simulink model "AcasXuClosedLoop_m_1_batch"  (Simulink 9.0)
settings: Solver=VariableStepAuto  StartTime=0.0  StopTime=20  RelTol=1e-3
values from neighbouring files (symbols this model uses):
  x1_i = randi([-20000,20000])    [Simulate_batch_m_1.m]
  x1_o = randi([-20000,20000])    [Simulate_batch_m_1.m]
  x2_i = randi([-20000,20000])    [Simulate_batch_m_1.m]
  x2_o = randi([-20000,20000])    [Simulate_batch_m_1.m]
  x3_i = rand*2*pi-pi    [Simulate_batch_m_1.m]
  x3_o = rand*2*pi-pi    [Simulate_batch_m_1.m]
libraries: neural x1560
(part 4 of 60)

== system AcasXuClosedLoop_m_1_batch/networks/AcasXu_4_1/Layer 1 ==
  "p{1}" : Inport  PortDimensions=5
      mask Display: e = 0.06;c1 = [120 230 180]/255;c2 = ([120 230 180]+0)/(256+128);c3 = ([120 230 180]+128)/(256+128);patch([0 1 1 0],[0 0 1 1],c1);patch([0 1 1 1-e 1-e 0+e],[0 0 1 1-e 0+e 0+e],c2);patch([0 0 1 1-e 0+e 0+e],[0 1 1 1-e 1-e 0+e],c3);plot([0 1 1 0 0],[0 0 1 1 0]);
  Delays 1 : Subsystem
      mask Display: e = 0.06;c1 = [120 150 230]/255;c2 = ([120 150 230]+0)/383;c3 = ([120 150 230]+128)/383;patch([0 1 1 0],[0 0 1 1],c1);patch([0 1 1 1-e 1-e 0+e],[0 0 1 1-e 0+e 0+e],c2);patch([0 0 1 1-e 0+e 0+e],[0 1 1 1-e 1-e 0+e],c3);plot([0 1 1 0 0],[0 0 1 1 0]);disp('0');
  "IW{1,1}" : Subsystem
      mask Display: e = 0.06;c1 = [120 150 230]/255;c2 = ([120 150 230]+0)/383;c3 = ([120 150 230]+128)/383;patch([0 1 1 0],[0 0 1 1],c1);patch([0 1 1 1-e 1-e 0+e],[0 0 1 1-e 0+e 0+e],c2);patch([0 0 1 1-e 0+e 0+e],[0 1 1 1-e 1-e 0+e],c3);plot([0 1 1 0 0],[0 0 1 1 0]);disp('W');
  "b{1}" : Constant  Value=[0.1348229999999999983995024877003743313252925872802734375;-0.0238180999999999983007814563507054117508232593536376953125;-0.11955000000000000348610029732299153693020343780517578125;-0.380141000000000006675549002466141246259212493896484375;-0.453220000000000011741718708435655571520328521728515625;-0.7901599999999999734967559561482630670070648193359375;-0.59201899999999996193622564533143304288387298583984375;-0.060997400000000000230837571280062547884881496429443359375;0.041191899999999996573318838954946841113269329071044921875;0.138994000000000006433964472307707183063030242919921875;0.5230390000000000316759951601852662861347198486328125;0.1683389999999999886437507257141987793147563934326171875;-0.2097199999999999897593028208575560711324214935302734375;-0.30047699999999999409538986583356745541095733642578125;-0.6601470000000000393214349969639442861080169677734375;0.33775800000000000267164068645797669887542724609375;-0.72938300000000000355981910615810193121433258056640625;-0.354287999999999991818100397722446359694004058837890625;0.330224000000000017518431150165270082652568817138671875;-0.01135309999999999965025754278258318663574755191802978515625;-0.0360036999999999995925037410415825434029102325439453125;-0.0244249999999999987954080182817051536403596401214599609375;0.2328119999999999911732828650201554410159587860107421875;0.1819800000000000028688162956314045004546642303466796875;0.03522630000000000205506722750214976258575916290283203125;0.45888200000000001210764821735210716724395751953125;-0.178321000000000007279510327862226404249668121337890625;0.055081100000000000893596308060295996256172657012939453125;-0.01507080000000000051529891464952015667222440242767333984375;-0.4580159999999999786979287819121964275836944580078125;-0.0500089999999999978985698589895037002861499786376953125;0.06709189999999999598490063590361387468874454498291015625;-0.323990999999999973457676105681457556784152984619140625;0.233887000000000011556977597138029523193836212158203125;0.1795219999999999871409528395815868861973285675048828125;-0.47103499999999998149036173344939015805721282958984375;0.11780400000000000593214366517713642679154872894287109375;-0.1605830000000000035154101851730956695973873138427734375;-0.02595340000000000146851419913218705914914608001708984375;-0.19649199999999999999289457264239899814128875732421875;0.09367630000000000400905975084242527373135089874267578125;-0.264062999999999992173371765602496452629566192626953125;-0.350741000000000024972024448288721032440662384033203125;-0.68448200000000003484501576167531311511993408203125;-0.2153859999999999941255879321033717133104801177978515625;0.60005299999999994753352439147420227527618408203125;0.276144000000000000571986902286880649626255035400390625;-0.57574000000000002952305067083216272294521331787109375;-0.5823739999999999472635181518853642046451568603515625;0.1123949999999999949107376551182824186980724334716796875]
      mask Display: e = 0.06;c1 = [120 150 230]/255;c2 = ([120 150 230]+0)/383;c3 = ([120 150 230]+128)/383;patch([0 1 1 0],[0 0 1 1],c1);patch([0 1 1 1-e 1-e 0+e],[0 0 1 1-e 0+e 0+e],c2);patch([0 0 1 1-e 0+e 0+e],[0 1 1 1-e 1-e 0+e],c3);plot([0 1 1 0 0],[0 0 1 1 0]);disp('b');
  netsum : Sum  Inputs=2  SaturateOnIntegerOverflow=on  InputSameDT=on  OutDataTypeStr=Inherit: Same as first input
      mask Display: e = 0.06;c1 = [120 150 230]/255;c2 = ([120 150 230]+0)/383;c3 = ([120 150 230]+128)/383;patch([0 1 1 0],[0 0 1 1],c1);patch([0 1 1 1-e 1-e 0+e],[0 0 1 1-e 0+e 0+e],c2);patch([0 0 1 1-e 0+e 0+e],[0 1 1 1-e 1-e 0+e],c3);plot([0 1 1 0 0],[0 0 1 1 0]);disp('+');
  poslin : neural/Transfer Functions/poslin
      settings: SourceProductName=Deep Learning Toolbox  SourceProductBaseCode=NN
  "a{1}" : Outport  InitialOutput=[0;0;0;0;0;0;0;0;0;0;0;0;0;0;0;0;0;0;0;0;0;0;0;0;0;0;0;0;0;0;0;0;0;0;0;0;0;0;0;0;0;0;0;0;0;0;0;0;0;0]
      mask Display: e = 0.06;c1 = [120 230 180]/255;c2 = ([120 230 180]+0)/(256+128);c3 = ([120 230 180]+128)/(256+128);patch([0 1 1 0],[0 0 1 1],c1);patch([0 1 1 1-e 1-e 0+e],[0 0 1 1-e 0+e 0+e],c2);patch([0 0 1 1-e 0+e 0+e],[0 1 1 1-e 1-e 0+e],c3);plot([0 1 1 0 0],[0 0 1 1 0]);
  wires:
  poslin/1 -> "a{1}"/1
  netsum/1 -> poslin/1
  "b{1}"/1 -> netsum/2
  "IW{1,1}"/1("iz{1,1}") -> netsum/1
  Delays 1/1("pd{1,1}") -> "IW{1,1}"/1("pd{1,1}")
  "p{1}"/1 -> Delays 1/1("p{1}")

== system AcasXuClosedLoop_m_1_batch/networks/AcasXu_4_1/Layer 2 ==
  "a{1} " : Inport  PortDimensions=50
      mask Display: e = 0.06;c1 = [120 230 180]/255;c2 = ([120 230 180]+0)/(256+128);c3 = ([120 230 180]+128)/(256+128);patch([0 1 1 0],[0 0 1 1],c1);patch([0 1 1 1-e 1-e 0+e],[0 0 1 1-e 0+e 0+e],c2);patch([0 0 1 1-e 0+e 0+e],[0 1 1 1-e 1-e 0+e],c3);plot([0 1 1 0 0],[0 0 1 1 0]);
  Delays 1 : Subsystem
      mask Display: e = 0.06;c1 = [120 150 230]/255;c2 = ([120 150 230]+0)/383;c3 = ([120 150 230]+128)/383;patch([0 1 1 0],[0 0 1 1],c1);patch([0 1 1 1-e 1-e 0+e],[0 0 1 1-e 0+e 0+e],c2);patch([0 0 1 1-e 0+e 0+e],[0 1 1 1-e 1-e 0+e],c3);plot([0 1 1 0 0],[0 0 1 1 0]);disp('0');
  "LW{2,1}" : Subsystem
      mask Display: e = 0.06;c1 = [120 150 230]/255;c2 = ([120 150 230]+0)/383;c3 = ([120 150 230]+128)/383;patch([0 1 1 0],[0 0 1 1],c1);patch([0 1 1 1-e 1-e 0+e],[0 0 1 1-e 0+e 0+e],c2);patch([0 0 1 1-e 0+e 0+e],[0 1 1 1-e 1-e 0+e],c3);plot([0 1 1 0 0],[0 0 1 1 0]);disp('W');
  "b{2}" : Constant  Value=[0.432821999999999984520826501466217450797557830810546875;-1.259370000000000100470742836478166282176971435546875;-0.45713700000000001555378048578859306871891021728515625;-0.65248300000000003517897084748256020247936248779296875;0.0116892000000000002846167745929051307030022144317626953125;0.9686099999999999710098563809879124164581298828125;-0.00109700000000000010176581799470341138658113777637481689453125;-0.01449650000000000070465855372958685620687901973724365234375;-0.01553530000000000015070167336261874879710376262664794921875;-0.1711300000000000043343106881366111338138580322265625;1.346410000000000106723518911167047917842864990234375;-0.0236382000000000015715873047383865923620760440826416015625;1.136740000000000083701934272539801895618438720703125;-0.98688699999999995871036162498057819902896881103515625;-0.202730999999999994543031789362430572509765625;0.28883599999999998164668113531661219894886016845703125;-0.18953900000000001302424834648263640701770782470703125;-0.67643200000000003324629460621508769690990447998046875;-0.77303200000000005243094847173779271543025970458984375;1.2642599999999999393907046396634541451930999755859375;-0.51136499999999995846877709482214413583278656005859375;1.3603799999999999226218960757250897586345672607421875;-0.0432717999999999991533883303418406285345554351806640625;-0.273573999999999983856469043530523777008056640625;0.3089749999999999996447286321199499070644378662109375;-0.05264359999999999872866141004124074243009090423583984375;1.1708000000000000628830321147688664495944976806640625;-0.01064329999999999963600227914639617665670812129974365234375;-0.50644199999999994776800349427503533661365509033203125;0.1564000000000000112354570092065841890871524810791015625;0.1855920000000000069650951672883820720016956329345703125;-0.0237028999999999989700683045157347805798053741455078125;-0.95172800000000001841016228354419581592082977294921875;-0.322857000000000005091038701721117831766605377197265625;-0.382485000000000019415580254644737578928470611572265625;-0.1345760000000000011777245845223660580813884735107421875;-0.01455150000000000019839685450051547377370297908782958984375;-0.269760999999999973031350464225397445261478424072265625;-0.88523399999999996534683077697991393506526947021484375;9.9680700000000002614607141904201625948189757764339447021484375e-05;-0.0100038999999999995982324918486483511514961719512939453125;1.2512399999999999078426071719150058925151824951171875;0.171111999999999986332710477654472924768924713134765625;-0.409106000000000025185187269016751088201999664306640625;-0.6994439999999999546531626037904061377048492431640625;0.444589999999999985202947527795913629233837127685546875;0.535841000000000011738165994756855070590972900390625;0.04694199999999999761701729994456400163471698760986328125;0.2113869999999999915729631538852117955684661865234375;0.58456699999999994776800349427503533661365509033203125]
      mask Display: e = 0.06;c1 = [120 150 230]/255;c2 = ([120 150 230]+0)/383;c3 = ([120 150 230]+128)/383;patch([0 1 1 0],[0 0 1 1],c1);patch([0 1 1 1-e 1-e 0+e],[0 0 1 1-e 0+e 0+e],c2);patch([0 0 1 1-e 0+e 0+e],[0 1 1 1-e 1-e 0+e],c3);plot([0 1 1 0 0],[0 0 1 1 0]);disp('b');
  netsum : Sum  Inputs=2  SaturateOnIntegerOverflow=on  InputSameDT=on  OutDataTypeStr=Inherit: Same as first input
      mask Display: e = 0.06;c1 = [120 150 230]/255;c2 = ([120 150 230]+0)/383;c3 = ([120 150 230]+128)/383;patch([0 1 1 0],[0 0 1 1],c1);patch([0 1 1 1-e 1-e 0+e],[0 0 1 1-e 0+e 0+e],c2);patch([0 0 1 1-e 0+e 0+e],[0 1 1 1-e 1-e 0+e],c3);plot([0 1 1 0 0],[0 0 1 1 0]);disp('+');
  poslin : neural/Transfer Functions/poslin
      settings: SourceProductName=Deep Learning Toolbox  SourceProductBaseCode=NN
  "a{2}" : Outport  InitialOutput=[0;0;0;0;0;0;0;0;0;0;0;0;0;0;0;0;0;0;0;0;0;0;0;0;0;0;0;0;0;0;0;0;0;0;0;0;0;0;0;0;0;0;0;0;0;0;0;0;0;0]
      mask Display: e = 0.06;c1 = [120 230 180]/255;c2 = ([120 230 180]+0)/(256+128);c3 = ([120 230 180]+128)/(256+128);patch([0 1 1 0],[0 0 1 1],c1);patch([0 1 1 1-e 1-e 0+e],[0 0 1 1-e 0+e 0+e],c2);patch([0 0 1 1-e 0+e 0+e],[0 1 1 1-e 1-e 0+e],c3);plot([0 1 1 0 0],[0 0 1 1 0]);
  wires:
  poslin/1 -> "a{2}"/1
  netsum/1 -> poslin/1
  "b{2}"/1 -> netsum/2
  "LW{2,1}"/1("lz{2,1}") -> netsum/1
  Delays 1/1("pd{2,1}") -> "LW{2,1}"/1("ad{2,1}")
  "a{1} "/1 -> Delays 1/1("p{2}")

== system AcasXuClosedLoop_m_1_batch/networks/AcasXu_4_1/Layer 3 ==
  "a{2} " : Inport  PortDimensions=50
      mask Display: e = 0.06;c1 = [120 230 180]/255;c2 = ([120 230 180]+0)/(256+128);c3 = ([120 230 180]+128)/(256+128);patch([0 1 1 0],[0 0 1 1],c1);patch([0 1 1 1-e 1-e 0+e],[0 0 1 1-e 0+e 0+e],c2);patch([0 0 1 1-e 0+e 0+e],[0 1 1 1-e 1-e 0+e],c3);plot([0 1 1 0 0],[0 0 1 1 0]);
  Delays 1 : Subsystem
      mask Display: e = 0.06;c1 = [120 150 230]/255;c2 = ([120 150 230]+0)/383;c3 = ([120 150 230]+128)/383;patch([0 1 1 0],[0 0 1 1],c1);patch([0 1 1 1-e 1-e 0+e],[0 0 1 1-e 0+e 0+e],c2);patch([0 0 1 1-e 0+e 0+e],[0 1 1 1-e 1-e 0+e],c3);plot([0 1 1 0 0],[0 0 1 1 0]);disp('0');
  "LW{3,2}" : Subsystem
      mask Display: e = 0.06;c1 = [120 150 230]/255;c2 = ([120 150 230]+0)/383;c3 = ([120 150 230]+128)/383;patch([0 1 1 0],[0 0 1 1],c1);patch([0 1 1 1-e 1-e 0+e],[0 0 1 1-e 0+e 0+e],c2);patch([0 0 1 1-e 0+e 0+e],[0 1 1 1-e 1-e 0+e],c3);plot([0 1 1 0 0],[0 0 1 1 0]);disp('W');
  "b{3}" : Constant  Value=[0.75966199999999994840749195645912550389766693115234375;-0.838961999999999985533349899924360215663909912109375;-1.1200300000000000810729261502274312078952789306640625;-0.51097300000000001052313791660708375275135040283203125;-0.135124999999999995115018691649311222136020660400390625;-0.82995099999999999429434183184639550745487213134765625;-0.7141610000000000457731630376656539738178253173828125;1.510499999999999953814722175593487918376922607421875;0.282934999999999992059684927880880422890186309814453125;-0.294370999999999993779198348420322872698307037353515625;-0.455872999999999972686737237381748855113983154296875;-0.7350980000000000291748847303097136318683624267578125;-0.36855599999999999472066747330245561897754669189453125;-0.141824000000000005616840326183591969311237335205078125;-0.9845960000000000267306177192949689924716949462890625;-0.1444709999999999883169010672645526938140392303466796875;0.63247799999999998465938233493943698704242706298828125;-0.6318770000000000219841922444174997508525848388671875;-0.00854197999999999936504568864847897202707827091217041015625;0.61706099999999997063326873103505931794643402099609375;-1.305309999999999970299313645227812230587005615234375;0.65445100000000000495248286824789829552173614501953125;-0.8968920000000000225526264330255798995494842529296875;0.022811299999999999632560587770058191381394863128662109375;-0.347494000000000025085711286010337062180042266845703125;-0.138658000000000003471001264188089407980442047119140625;-0.81998499999999996390442902338691055774688720703125;-1.0180700000000000304822833641082979738712310791015625;-0.88269600000000003614530896811629645526409149169921875;-1.585620000000000029416469260468147695064544677734375;-1.1131299999999999528199623455293476581573486328125;-0.888298999999999949750417727045714855194091796875;-1.0311099999999999710098563809879124164581298828125;-0.48253800000000002246025587737676687538623809814453125;-0.363474000000000019294787989565520547330379486083984375;-0.670150000000000023447910280083306133747100830078125;-0.645361999999999991217691786005161702632904052734375;0.36204300000000000370192765331012196838855743408203125;-1.3692699999999999871391764827421866357326507568359375;-2.15331999999999990080823408789001405239105224609375;0.321091999999999988535392958510783500969409942626953125;1.4917700000000000404298816647497005760669708251953125;0.1303020000000000011564083024495630525052547454833984375;-0.7060370000000000256790144703700207173824310302734375;1.1547400000000000996891458271420560777187347412109375;-0.73546699999999998187405481075984425842761993408203125;-0.207056999999999991057819670459139160811901092529296875;-0.95738800000000001677591399129596538841724395751953125;-1.3000700000000000589039927945123054087162017822265625;-0.1655649999999999899547020731915836222469806671142578125]
      mask Display: e = 0.06;c1 = [120 150 230]/255;c2 = ([120 150 230]+0)/383;c3 = ([120 150 230]+128)/383;patch([0 1 1 0],[0 0 1 1],c1);patch([0 1 1 1-e 1-e 0+e],[0 0 1 1-e 0+e 0+e],c2);patch([0 0 1 1-e 0+e 0+e],[0 1 1 1-e 1-e 0+e],c3);plot([0 1 1 0 0],[0 0 1 1 0]);disp('b');
  netsum : Sum  Inputs=2  SaturateOnIntegerOverflow=on  InputSameDT=on  OutDataTypeStr=Inherit: Same as first input
      mask Display: e = 0.06;c1 = [120 150 230]/255;c2 = ([120 150 230]+0)/383;c3 = ([120 150 230]+128)/383;patch([0 1 1 0],[0 0 1 1],c1);patch([0 1 1 1-e 1-e 0+e],[0 0 1 1-e 0+e 0+e],c2);patch([0 0 1 1-e 0+e 0+e],[0 1 1 1-e 1-e 0+e],c3);plot([0 1 1 0 0],[0 0 1 1 0]);disp('+');
  poslin : neural/Transfer Functions/poslin
      settings: SourceProductName=Deep Learning Toolbox  SourceProductBaseCode=NN
  "a{3}" : Outport  InitialOutput=[0;0;0;0;0;0;0;0;0;0;0;0;0;0;0;0;0;0;0;0;0;0;0;0;0;0;0;0;0;0;0;0;0;0;0;0;0;0;0;0;0;0;0;0;0;0;0;0;0;0]
      mask Display: e = 0.06;c1 = [120 230 180]/255;c2 = ([120 230 180]+0)/(256+128);c3 = ([120 230 180]+128)/(256+128);patch([0 1 1 0],[0 0 1 1],c1);patch([0 1 1 1-e 1-e 0+e],[0 0 1 1-e 0+e 0+e],c2);patch([0 0 1 1-e 0+e 0+e],[0 1 1 1-e 1-e 0+e],c3);plot([0 1 1 0 0],[0 0 1 1 0]);
  wires:
  poslin/1 -> "a{3}"/1
  netsum/1 -> poslin/1
  "b{3}"/1 -> netsum/2
  "LW{3,2}"/1("lz{3,2}") -> netsum/1
  Delays 1/1("pd{3,2}") -> "LW{3,2}"/1("ad{3,2}")
  "a{2} "/1 -> Delays 1/1("p{3}")

== system AcasXuClosedLoop_m_1_batch/networks/AcasXu_4_1/Layer 4 ==
  "a{3} " : Inport  PortDimensions=50
      mask Display: e = 0.06;c1 = [120 230 180]/255;c2 = ([120 230 180]+0)/(256+128);c3 = ([120 230 180]+128)/(256+128);patch([0 1 1 0],[0 0 1 1],c1);patch([0 1 1 1-e 1-e 0+e],[0 0 1 1-e 0+e 0+e],c2);patch([0 0 1 1-e 0+e 0+e],[0 1 1 1-e 1-e 0+e],c3);plot([0 1 1 0 0],[0 0 1 1 0]);
  Delays 1 : Subsystem
      mask Display: e = 0.06;c1 = [120 150 230]/255;c2 = ([120 150 230]+0)/383;c3 = ([120 150 230]+128)/383;patch([0 1 1 0],[0 0 1 1],c1);patch([0 1 1 1-e 1-e 0+e],[0 0 1 1-e 0+e 0+e],c2);patch([0 0 1 1-e 0+e 0+e],[0 1 1 1-e 1-e 0+e],c3);plot([0 1 1 0 0],[0 0 1 1 0]);disp('0');
  "LW{4,3}" : Subsystem
      mask Display: e = 0.06;c1 = [120 150 230]/255;c2 = ([120 150 230]+0)/383;c3 = ([120 150 230]+128)/383;patch([0 1 1 0],[0 0 1 1],c1);patch([0 1 1 1-e 1-e 0+e],[0 0 1 1-e 0+e 0+e],c2);patch([0 0 1 1-e 0+e 0+e],[0 1 1 1-e 1-e 0+e],c3);plot([0 1 1 0 0],[0 0 1 1 0]);disp('W');
  "b{4}" : Constant  Value=[-0.6820079999999999476045786650502122938632965087890625;-3.08436999999999983401721692644059658050537109375;0.585555999999999965410779623198322951793670654296875;-0.5398359999999999825348595550167374312877655029296875;-0.55025299999999999212008106042048893868923187255859375;0.0256048000000000004761080418802521307952702045440673828125;-1.7290099999999999358379909608629532158374786376953125;-0.1298610000000000042064129956997931003570556640625;-0.8305789999999999562163566224626265466213226318359375;-0.196811999999999986954435371444560587406158447265625;-0.6160639999999999449897813974530436098575592041015625;0.3433990000000000097912788987741805613040924072265625;-1.597210000000000018616219676914624869823455810546875;0.1576450000000000073452355309200356714427471160888671875;0.88317800000000001858779796748422086238861083984375;-0.70011999999999996457944462235900573432445526123046875;-0.286934000000000022367885321727953851222991943359375;0.637162000000000006139089236967265605926513671875;1.2516700000000000603250782660325057804584503173828125;-0.01162849999999999994759747323769261129200458526611328125;-0.5578469999999999817674734003958292305469512939453125;-1.00337999999999993860910763032734394073486328125;1.05571999999999999175770426518283784389495849609375;-1.24138999999999999346300683100707828998565673828125;0.186377999999999988123278171769925393164157867431640625;0.0017374199999999999366462333227900671772658824920654296875;0.105161000000000004472866521609830670058727264404296875;0.54805800000000004512656914812396280467510223388671875;0.08856619999999999770512459917881642468273639678955078125;0.1579730000000000023074875343809253536164760589599609375;-0.0757977999999999985103471544789499603211879730224609375;1.2585900000000000975575176198617555201053619384765625;-1.461959999999999926245664028101600706577301025390625;0.62902299999999999879918277656543068587779998779296875;-0.7479839999999999822222207512822933495044708251953125;0.09964230000000000309068326487249578349292278289794921875;1.006110000000000059827698351000435650348663330078125;0.77711699999999994670218939063488505780696868896484375;0.325390000000000012558842854559770785272121429443359375;-1.7636499999999999399591388282715342938899993896484375;-0.53327899999999994751220810940139926970005035400390625;-0.77692799999999995197441648997482843697071075439453125;-0.73104299999999999837285713510937057435512542724609375;0.64756599999999997496757941917167045176029205322265625;-0.1434709999999999874287226475644274614751338958740234375;0.751273999999999997356781022972427308559417724609375;0.4473059999999999813979911778005771338939666748046875;1.2945800000000000640199004919850267469882965087890625;-0.55212600000000000566302560400799848139286041259765625;1.038089999999999957225327307241968810558319091796875]
      mask Display: e = 0.06;c1 = [120 150 230]/255;c2 = ([120 150 230]+0)/383;c3 = ([120 150 230]+128)/383;patch([0 1 1 0],[0 0 1 1],c1);patch([0 1 1 1-e 1-e 0+e],[0 0 1 1-e 0+e 0+e],c2);patch([0 0 1 1-e 0+e 0+e],[0 1 1 1-e 1-e 0+e],c3);plot([0 1 1 0 0],[0 0 1 1 0]);disp('b');
  netsum : Sum  Inputs=2  SaturateOnIntegerOverflow=on  InputSameDT=on  OutDataTypeStr=Inherit: Same as first input
      mask Display: e = 0.06;c1 = [120 150 230]/255;c2 = ([120 150 230]+0)/383;c3 = ([120 150 230]+128)/383;patch([0 1 1 0],[0 0 1 1],c1);patch([0 1 1 1-e 1-e 0+e],[0 0 1 1-e 0+e 0+e],c2);patch([0 0 1 1-e 0+e 0+e],[0 1 1 1-e 1-e 0+e],c3);plot([0 1 1 0 0],[0 0 1 1 0]);disp('+');
  poslin : neural/Transfer Functions/poslin
      settings: SourceProductName=Deep Learning Toolbox  SourceProductBaseCode=NN
  "a{4}" : Outport  InitialOutput=[0;0;0;0;0;0;0;0;0;0;0;0;0;0;0;0;0;0;0;0;0;0;0;0;0;0;0;0;0;0;0;0;0;0;0;0;0;0;0;0;0;0;0;0;0;0;0;0;0;0]
      mask Display: e = 0.06;c1 = [120 230 180]/255;c2 = ([120 230 180]+0)/(256+128);c3 = ([120 230 180]+128)/(256+128);patch([0 1 1 0],[0 0 1 1],c1);patch([0 1 1 1-e 1-e 0+e],[0 0 1 1-e 0+e 0+e],c2);patch([0 0 1 1-e 0+e 0+e],[0 1 1 1-e 1-e 0+e],c3);plot([0 1 1 0 0],[0 0 1 1 0]);
  wires:
  poslin/1 -> "a{4}"/1
  netsum/1 -> poslin/1
  "b{4}"/1 -> netsum/2
  "LW{4,3}"/1("lz{4,3}") -> netsum/1
  Delays 1/1("pd{4,3}") -> "LW{4,3}"/1("ad{4,3}")
  "a{3} "/1 -> Delays 1/1("p{4}")

== system AcasXuClosedLoop_m_1_batch/networks/AcasXu_4_1/Layer 5 ==
  "a{4} " : Inport  PortDimensions=50
      mask Display: e = 0.06;c1 = [120 230 180]/255;c2 = ([120 230 180]+0)/(256+128);c3 = ([120 230 180]+128)/(256+128);patch([0 1 1 0],[0 0 1 1],c1);patch([0 1 1 1-e 1-e 0+e],[0 0 1 1-e 0+e 0+e],c2);patch([0 0 1 1-e 0+e 0+e],[0 1 1 1-e 1-e 0+e],c3);plot([0 1 1 0 0],[0 0 1 1 0]);
  Delays 1 : Subsystem
      mask Display: e = 0.06;c1 = [120 150 230]/255;c2 = ([120 150 230]+0)/383;c3 = ([120 150 230]+128)/383;patch([0 1 1 0],[0 0 1 1],c1);patch([0 1 1 1-e 1-e 0+e],[0 0 1 1-e 0+e 0+e],c2);patch([0 0 1 1-e 0+e 0+e],[0 1 1 1-e 1-e 0+e],c3);plot([0 1 1 0 0],[0 0 1 1 0]);disp('0');
  "LW{5,4}" : Subsystem
      mask Display: e = 0.06;c1 = [120 150 230]/255;c2 = ([120 150 230]+0)/383;c3 = ([120 150 230]+128)/383;patch([0 1 1 0],[0 0 1 1],c1);patch([0 1 1 1-e 1-e 0+e],[0 0 1 1-e 0+e 0+e],c2);patch([0 0 1 1-e 0+e 0+e],[0 1 1 1-e 1-e 0+e],c3);plot([0 1 1 0 0],[0 0 1 1 0]);disp('W');
  "b{5}" : Constant  Value=[-1.148800000000000043343106881366111338138580322265625;-1.268780000000000018900436771218664944171905517578125;-0.448139999999999982804865794605575501918792724609375;0.4046790000000000109281472759903408586978912353515625;1.01714999999999999857891452847979962825775146484375;0.305279000000000022563284574061981402337551116943359375;0.08392149999999999610000855909675010479986667633056640625;0.52215100000000003177547114319168031215667724609375;-1.074980000000000046611603465862572193145751953125;-1.4273800000000000931521526581491343677043914794921875;0.52887099999999998001243284306838177144527435302734375;-0.284766999999999992354560163221322000026702880859375;0.462851999999999985657694878682377748191356658935546875;-1.434930000000000038795633372501470148563385009765625;1.021269999999999900097691352129913866519927978515625;1.4297100000000000363797880709171295166015625;-0.55277399999999998758681840627104975283145904541015625;-1.43315999999999998948396751075051724910736083984375;0.64438099999999998157562686174060218036174774169921875;1.3235900000000000442668124378542415797710418701171875;-0.356341000000000018843593352357856929302215576171875;-1.3253399999999999625543978254427202045917510986328125;0.47899700000000000610356210017926059663295745849609375;0.09044280000000000374615893861118820495903491973876953125;-0.1897939999999999904911618386904592625796794891357421875;0.081688700000000002976463520099059678614139556884765625;-0.1393810000000000048903103788688895292580127716064453125;0.79328699999999996439470351106137968599796295166015625;0.853005000000000013216094885137863457202911376953125;0.8116689999999999738378164693131111562252044677734375;0.1342210000000000069686478809671825729310512542724609375;-0.89552500000000001545430450278217904269695281982421875;0.41882399999999997408650642682914622128009796142578125;0.5555499999999999882760448599583469331264495849609375;0.2302760000000000084607876260633929632604122161865234375;1.2937499999999999555910790149937383830547332763671875;0.65880199999999999871391764827421866357326507568359375;0.387861999999999984556353638254222460091114044189453125;-0.1655999999999999972022379779446055181324481964111328125;1.0075700000000000766675611885148100554943084716796875;0.194539999999999990709653729936690069735050201416015625;-1.03254000000000001335820343228988349437713623046875;-0.144120999999999999108268866621074266731739044189453125;0.42739700000000002688693712116219103336334228515625;-1.1556599999999999095479097377392463386058807373046875;1.261789999999999967172925607883371412754058837890625;-0.282252000000000002888356220864807255566120147705078125;-0.00985550999999999961798113901068063569255173206329345703125;0.88892300000000001869437937784823589026927947998046875;2.444459999999999855191390452091582119464874267578125]
      mask Display: e = 0.06;c1 = [120 150 230]/255;c2 = ([120 150 230]+0)/383;c3 = ([120 150 230]+128)/383;patch([0 1 1 0],[0 0 1 1],c1);patch([0 1 1 1-e 1-e 0+e],[0 0 1 1-e 0+e 0+e],c2);patch([0 0 1 1-e 0+e 0+e],[0 1 1 1-e 1-e 0+e],c3);plot([0 1 1 0 0],[0 0 1 1 0]);disp('b');
  netsum : Sum  Inputs=2  SaturateOnIntegerOverflow=on  InputSameDT=on  OutDataTypeStr=Inherit: Same as first input
      mask Display: e = 0.06;c1 = [120 150 230]/255;c2 = ([120 150 230]+0)/383;c3 = ([120 150 230]+128)/383;patch([0 1 1 0],[0 0 1 1],c1);patch([0 1 1 1-e 1-e 0+e],[0 0 1 1-e 0+e 0+e],c2);patch([0 0 1 1-e 0+e 0+e],[0 1 1 1-e 1-e 0+e],c3);plot([0 1 1 0 0],[0 0 1 1 0]);disp('+');
  poslin : neural/Transfer Functions/poslin
      settings: SourceProductName=Deep Learning Toolbox  SourceProductBaseCode=NN
  "a{5}" : Outport  InitialOutput=[0;0;0;0;0;0;0;0;0;0;0;0;0;0;0;0;0;0;0;0;0;0;0;0;0;0;0;0;0;0;0;0;0;0;0;0;0;0;0;0;0;0;0;0;0;0;0;0;0;0]
      mask Display: e = 0.06;c1 = [120 230 180]/255;c2 = ([120 230 180]+0)/(256+128);c3 = ([120 230 180]+128)/(256+128);patch([0 1 1 0],[0 0 1 1],c1);patch([0 1 1 1-e 1-e 0+e],[0 0 1 1-e 0+e 0+e],c2);patch([0 0 1 1-e 0+e 0+e],[0 1 1 1-e 1-e 0+e],c3);plot([0 1 1 0 0],[0 0 1 1 0]);
  wires:
  poslin/1 -> "a{5}"/1
  netsum/1 -> poslin/1
  "b{5}"/1 -> netsum/2
  "LW{5,4}"/1("lz{5,4}") -> netsum/1
  Delays 1/1("pd{5,4}") -> "LW{5,4}"/1("ad{5,4}")
  "a{4} "/1 -> Delays 1/1("p{5}")

== system AcasXuClosedLoop_m_1_batch/networks/AcasXu_4_1/Layer 6 ==
  "a{5} " : Inport  PortDimensions=50
      mask Display: e = 0.06;c1 = [120 230 180]/255;c2 = ([120 230 180]+0)/(256+128);c3 = ([120 230 180]+128)/(256+128);patch([0 1 1 0],[0 0 1 1],c1);patch([0 1 1 1-e 1-e 0+e],[0 0 1 1-e 0+e 0+e],c2);patch([0 0 1 1-e 0+e 0+e],[0 1 1 1-e 1-e 0+e],c3);plot([0 1 1 0 0],[0 0 1 1 0]);
  Delays 1 : Subsystem
      mask Display: e = 0.06;c1 = [120 150 230]/255;c2 = ([120 150 230]+0)/383;c3 = ([120 150 230]+128)/383;patch([0 1 1 0],[0 0 1 1],c1);patch([0 1 1 1-e 1-e 0+e],[0 0 1 1-e 0+e 0+e],c2);patch([0 0 1 1-e 0+e 0+e],[0 1 1 1-e 1-e 0+e],c3);plot([0 1 1 0 0],[0 0 1 1 0]);disp('0');
  "LW{6,5}" : Subsystem
      mask Display: e = 0.06;c1 = [120 150 230]/255;c2 = ([120 150 230]+0)/383;c3 = ([120 150 230]+128)/383;patch([0 1 1 0],[0 0 1 1],c1);patch([0 1 1 1-e 1-e 0+e],[0 0 1 1-e 0+e 0+e],c2);patch([0 0 1 1-e 0+e 0+e],[0 1 1 1-e 1-e 0+e],c3);plot([0 1 1 0 0],[0 0 1 1 0]);disp('W');
  "b{6}" : Constant  Value=[-2.6473800000000000665068000671453773975372314453125;-0.10213899999999999368416325751240947283804416656494140625;0.09831600000000000061373128801278653554618358612060546875;0.2058689999999999964419572506812983192503452301025390625;-0.0282583999999999994912069922747832606546580791473388671875;0.196272000000000002017941369558684527873992919921875;-0.4957759999999999944719775157864205539226531982421875;-0.031972300000000002107025665054607088677585124969482421875;0.03432000000000000328181926079196273349225521087646484375;-0.005902010000000000068676175857262933277525007724761962890625;-0.08618030000000000134807720542085007764399051666259765625;-0.08027869999999999450768228825836558826267719268798828125;0.1025480000000000002646771690706373192369937896728515625;-0.09591170000000000250839349291709368117153644561767578125;-1.2270700000000001050892706189188174903392791748046875;0.14760200000000001097077984013594686985015869140625;-0.00941825999999999947942530553746109944768249988555908203125;-0.8689810000000000034248159863636828958988189697265625;-0.419634000000000007002398660915787331759929656982421875;-1.061059999999999892139612711616791784763336181640625;-0.234507999999999994233945699306786991655826568603515625;0.57218599999999997240962557043530978262424468994140625;0.3316470000000000251105802817619405686855316162109375;-1.96290999999999993264054864994250237941741943359375;-0.4404660000000000241726638705586083233356475830078125;-0.20329900000000000748201500755385495722293853759765625;-0.132805000000000006377121053446899168193340301513671875;-0.03263760000000000272013522817360353656113147735595703125;-0.472158000000000022122748077890719287097454071044921875;-0.54876899999999995127808460892993025481700897216796875;-1.289099999999999912603243501507677137851715087890625;0.037761700000000002208633276268301415257155895233154296875;0.2109080000000000121307408562643104232847690582275390625;0.41221600000000002683719912965898402035236358642578125;0.1534149999999999958166796432124101556837558746337890625;-0.598470999999999975216269376687705516815185546875;-0.5960170000000000189999127542250789701938629150390625;-2.02934999999999998721023075631819665431976318359375;0.36855599999999999472066747330245561897754669189453125;0.176721999999999990205168387547018937766551971435546875;-0.72194700000000000539301936441916041076183319091796875;0.30531099999999999905497816143906675279140472412109375;0.3467620000000000146656020660884678363800048828125;-0.09170309999999999572839470829421770758926868438720703125;-2.207030000000000047322146201622672379016876220703125;-0.932763000000000008782308213994838297367095947265625;-0.377265000000000016999734953060396946966648101806640625;-1.375010000000000065512040237081237137317657470703125;-1.0118899999999999561595132036018185317516326904296875;0.2037650000000000016786572132332366891205310821533203125]
      mask Display: e = 0.06;c1 = [120 150 230]/255;c2 = ([120 150 230]+0)/383;c3 = ([120 150 230]+128)/383;patch([0 1 1 0],[0 0 1 1],c1);patch([0 1 1 1-e 1-e 0+e],[0 0 1 1-e 0+e 0+e],c2);patch([0 0 1 1-e 0+e 0+e],[0 1 1 1-e 1-e 0+e],c3);plot([0 1 1 0 0],[0 0 1 1 0]);disp('b');
  netsum : Sum  Inputs=2  SaturateOnIntegerOverflow=on  InputSameDT=on  OutDataTypeStr=Inherit: Same as first input
      mask Display: e = 0.06;c1 = [120 150 230]/255;c2 = ([120 150 230]+0)/383;c3 = ([120 150 230]+128)/383;patch([0 1 1 0],[0 0 1 1],c1);patch([0 1 1 1-e 1-e 0+e],[0 0 1 1-e 0+e 0+e],c2);patch([0 0 1 1-e 0+e 0+e],[0 1 1 1-e 1-e 0+e],c3);plot([0 1 1 0 0],[0 0 1 1 0]);disp('+');
  poslin : neural/Transfer Functions/poslin
      settings: SourceProductName=Deep Learning Toolbox  SourceProductBaseCode=NN
  "a{6}" : Outport  InitialOutput=[0;0;0;0;0;0;0;0;0;0;0;0;0;0;0;0;0;0;0;0;0;0;0;0;0;0;0;0;0;0;0;0;0;0;0;0;0;0;0;0;0;0;0;0;0;0;0;0;0;0]
      mask Display: e = 0.06;c1 = [120 230 180]/255;c2 = ([120 230 180]+0)/(256+128);c3 = ([120 230 180]+128)/(256+128);patch([0 1 1 0],[0 0 1 1],c1);patch([0 1 1 1-e 1-e 0+e],[0 0 1 1-e 0+e 0+e],c2);patch([0 0 1 1-e 0+e 0+e],[0 1 1 1-e 1-e 0+e],c3);plot([0 1 1 0 0],[0 0 1 1 0]);
  wires:
  poslin/1 -> "a{6}"/1
  netsum/1 -> poslin/1
  "b{6}"/1 -> netsum/2
  "LW{6,5}"/1("lz{6,5}") -> netsum/1
  Delays 1/1("pd{6,5}") -> "LW{6,5}"/1("ad{6,5}")
  "a{5} "/1 -> Delays 1/1("p{6}")

== system AcasXuClosedLoop_m_1_batch/networks/AcasXu_4_1/Layer 7 ==
  "a{6} " : Inport  PortDimensions=50
      mask Display: e = 0.06;c1 = [120 230 180]/255;c2 = ([120 230 180]+0)/(256+128);c3 = ([120 230 180]+128)/(256+128);patch([0 1 1 0],[0 0 1 1],c1);patch([0 1 1 1-e 1-e 0+e],[0 0 1 1-e 0+e 0+e],c2);patch([0 0 1 1-e 0+e 0+e],[0 1 1 1-e 1-e 0+e],c3);plot([0 1 1 0 0],[0 0 1 1 0]);
  Delays 1 : Subsystem
      mask Display: e = 0.06;c1 = [120 150 230]/255;c2 = ([120 150 230]+0)/383;c3 = ([120 150 230]+128)/383;patch([0 1 1 0],[0 0 1 1],c1);patch([0 1 1 1-e 1-e 0+e],[0 0 1 1-e 0+e 0+e],c2);patch([0 0 1 1-e 0+e 0+e],[0 1 1 1-e 1-e 0+e],c3);plot([0 1 1 0 0],[0 0 1 1 0]);disp('0');
  "LW{7,6}" : Subsystem
      mask Display: e = 0.06;c1 = [120 150 230]/255;c2 = ([120 150 230]+0)/383;c3 = ([120 150 230]+128)/383;patch([0 1 1 0],[0 0 1 1],c1);patch([0 1 1 1-e 1-e 0+e],[0 0 1 1-e 0+e 0+e],c2);patch([0 0 1 1-e 0+e 0+e],[0 1 1 1-e 1-e 0+e],c3);plot([0 1 1 0 0],[0 0 1 1 0]);disp('W');
  "b{7}" : Constant  Value=[-0.020869800000000000850253201178929884918034076690673828125;-0.01825859999999999983000265046939603053033351898193359375;0.0181765000000000016833201499366623465903103351593017578125;-0.018426800000000000123723253864227444864809513092041015625;0.01793809999999999849062959356160718016326427459716796875]
      mask Display: e = 0.06;c1 = [120 150 230]/255;c2 = ([120 150 230]+0)/383;c3 = ([120 150 230]+128)/383;patch([0 1 1 0],[0 0 1 1],c1);patch([0 1 1 1-e 1-e 0+e],[0 0 1 1-e 0+e 0+e],c2);patch([0 0 1 1-e 0+e 0+e],[0 1 1 1-e 1-e 0+e],c3);plot([0 1 1 0 0],[0 0 1 1 0]);disp('b');
  netsum : Sum  Inputs=2  SaturateOnIntegerOverflow=on  InputSameDT=on  OutDataTypeStr=Inherit: Same as first input
      mask Display: e = 0.06;c1 = [120 150 230]/255;c2 = ([120 150 230]+0)/383;c3 = ([120 150 230]+128)/383;patch([0 1 1 0],[0 0 1 1],c1);patch([0 1 1 1-e 1-e 0+e],[0 0 1 1-e 0+e 0+e],c2);patch([0 0 1 1-e 0+e 0+e],[0 1 1 1-e 1-e 0+e],c3);plot([0 1 1 0 0],[0 0 1 1 0]);disp('+');
  purelin : neural/Transfer Functions/purelin
      settings: SourceProductName=Deep Learning Toolbox
  "a{7}" : Outport  InitialOutput=[0;0;0;0;0]
      mask Display: e = 0.06;c1 = [120 230 180]/255;c2 = ([120 230 180]+0)/(256+128);c3 = ([120 230 180]+128)/(256+128);patch([0 1 1 0],[0 0 1 1],c1);patch([0 1 1 1-e 1-e 0+e],[0 0 1 1-e 0+e 0+e],c2);patch([0 0 1 1-e 0+e 0+e],[0 1 1 1-e 1-e 0+e],c3);plot([0 1 1 0 0],[0 0 1 1 0]);
  wires:
  purelin/1 -> "a{7}"/1
  netsum/1 -> purelin/1
  "b{7}"/1 -> netsum/2
  "LW{7,6}"/1("lz{7,6}") -> netsum/1
  Delays 1/1("pd{7,6}") -> "LW{7,6}"/1("ad{7,6}")
  "a{6} "/1 -> Delays 1/1("p{7}")

== system AcasXuClosedLoop_m_1_batch/networks/AcasXu_4_1/Process Input 1 ==
  x : Inport  PortDimensions=5
      mask Display: e = 0.06;c1 = [120 230 180]/255;c2 = ([120 230 180]+0)/(256+128);c3 = ([120 230 180]+128)/(256+128);patch([0 1 1 0],[0 0 1 1],c1);patch([0 1 1 1-e 1-e 0+e],[0 0 1 1-e 0+e 0+e],c2);patch([0 0 1 1-e 0+e 0+e],[0 1 1 1-e 1-e 0+e],c3);plot([0 1 1 0 0],[0 0 1 1 0]);
  p : Outport  PortDimensions=5
      mask Display: e = 0.06;c1 = [120 230 180]/255;c2 = ([120 230 180]+0)/(256+128);c3 = ([120 230 180]+128)/(256+128);patch([0 1 1 0],[0 0 1 1],c1);patch([0 1 1 1-e 1-e 0+e],[0 0 1 1-e 0+e 0+e],c2);patch([0 0 1 1-e 0+e 0+e],[0 1 1 1-e 1-e 0+e],c3);plot([0 1 1 0 0],[0 0 1 1 0]);
  wires:
  x/1 -> p/1

== system AcasXuClosedLoop_m_1_batch/networks/AcasXu_4_1/Process Output 1 ==
  a : Inport  PortDimensions=5
      mask Display: e = 0.06;c1 = [120 230 180]/255;c2 = ([120 230 180]+0)/(256+128);c3 = ([120 230 180]+128)/(256+128);patch([0 1 1 0],[0 0 1 1],c1);patch([0 1 1 1-e 1-e 0+e],[0 0 1 1-e 0+e 0+e],c2);patch([0 0 1 1-e 0+e 0+e],[0 1 1 1-e 1-e 0+e],c3);plot([0 1 1 0 0],[0 0 1 1 0]);
  y : Outport  PortDimensions=5
      mask Display: e = 0.06;c1 = [120 230 180]/255;c2 = ([120 230 180]+0)/(256+128);c3 = ([120 230 180]+128)/(256+128);patch([0 1 1 0],[0 0 1 1],c1);patch([0 1 1 1-e 1-e 0+e],[0 0 1 1-e 0+e 0+e],c2);patch([0 0 1 1-e 0+e 0+e],[0 1 1 1-e 1-e 0+e],c3);plot([0 1 1 0 0],[0 0 1 1 0]);
  wires:
  a/1 -> y/1

== system AcasXuClosedLoop_m_1_batch/networks/AcasXu_5_1/Layer 1 ==
  "p{1}" : Inport  PortDimensions=5
      mask Display: e = 0.06;c1 = [120 230 180]/255;c2 = ([120 230 180]+0)/(256+128);c3 = ([120 230 180]+128)/(256+128);patch([0 1 1 0],[0 0 1 1],c1);patch([0 1 1 1-e 1-e 0+e],[0 0 1 1-e 0+e 0+e],c2);patch([0 0 1 1-e 0+e 0+e],[0 1 1 1-e 1-e 0+e],c3);plot([0 1 1 0 0],[0 0 1 1 0]);
  Delays 1 : Subsystem
      mask Display: e = 0.06;c1 = [120 150 230]/255;c2 = ([120 150 230]+0)/383;c3 = ([120 150 230]+128)/383;patch([0 1 1 0],[0 0 1 1],c1);patch([0 1 1 1-e 1-e 0+e],[0 0 1 1-e 0+e 0+e],c2);patch([0 0 1 1-e 0+e 0+e],[0 1 1 1-e 1-e 0+e],c3);plot([0 1 1 0 0],[0 0 1 1 0]);disp('0');
  "IW{1,1}" : Subsystem
      mask Display: e = 0.06;c1 = [120 150 230]/255;c2 = ([120 150 230]+0)/383;c3 = ([120 150 230]+128)/383;patch([0 1 1 0],[0 0 1 1],c1);patch([0 1 1 1-e 1-e 0+e],[0 0 1 1-e 0+e 0+e],c2);patch([0 0 1 1-e 0+e 0+e],[0 1 1 1-e 1-e 0+e],c3);plot([0 1 1 0 0],[0 0 1 1 0]);disp('W');
  "b{1}" : Constant  Value=[0.27264399999999999746336243333644233644008636474609375;-0.7264909999999999978825826474349014461040496826171875;-0.77816899999999999959499064061674289405345916748046875;0.2486210000000000086561868783974205143749713897705078125;0.1726000000000000034194869158454821445047855377197265625;0.031532100000000000405986355644927243702113628387451171875;-0.084186499999999997445598864942439831793308258056640625;0.070256299999999993755039895404479466378688812255859375;-0.264100999999999974665598756473627872765064239501953125;-0.005442909999999999588748966772300263983197510242462158203125;0.282386999999999999122479721336276270449161529541015625;-0.6244279999999999830606611794792115688323974609375;-0.6145469999999999544115780736319720745086669921875;-0.0305731999999999982942089360449244850315153598785400390625;0.251867000000000007542411140093463473021984100341796875;-0.719573000000000018161472326028160750865936279296875;-0.408038999999999985046628125928691588342189788818359375;0.19076999999999999513278226004331372678279876708984375;-0.458693999999999990624388601645478047430515289306640625;0.11665899999999999880895273918213206343352794647216796875;0.021026099999999998957367353114022989757359027862548828125;-0.2186200000000000087663210024402360431849956512451171875;0.039987099999999997645971916426788084208965301513671875;-0.7091380000000000460858018414000980556011199951171875;-0.08869570000000000231654695426186663098633289337158203125;0.01514509999999999988185006571939084096811711788177490234375;-0.5971290000000000208757455766317434608936309814453125;-0.373078999999999993963939459717948921024799346923828125;-0.2486629999999999951487694715979159809648990631103515625;-0.45685100000000000708411107552819885313510894775390625;-0.0294940999999999987901677656054744147695600986480712890625;-0.5039919999999999955520024741417728364467620849609375;-0.450427999999999995051069845430902205407619476318359375;-0.383678000000000019031887177334283478558063507080078125;-0.00826648999999999960219820849260941031388938426971435546875;0.52696200000000004148859034103224985301494598388671875;-0.54082600000000002893330020015127956867218017578125;-0.0591807000000000027473134878164273686707019805908203125;-0.257616000000000011649348152786842547357082366943359375;-0.0716877999999999959701568741365917958319187164306640625;-0.7003369999999999873097067393246106803417205810546875;-0.176420999999999994489741084180423058569431304931640625;-0.348355000000000025739410602909629233181476593017578125;0.26371299999999997520916394933010451495647430419921875;-0.0024396199999999999830091468311366043053567409515380859375;0.10330999999999999905408998301936662755906581878662109375;0.0286719000000000001637801005927030928432941436767578125;-0.53494900000000000783728637543390505015850067138671875;-0.0248139999999999992519317260075695230625569820404052734375;0.440342999999999984428455945817404426634311676025390625]
      mask Display: e = 0.06;c1 = [120 150 230]/255;c2 = ([120 150 230]+0)/383;c3 = ([120 150 230]+128)/383;patch([0 1 1 0],[0 0 1 1],c1);patch([0 1 1 1-e 1-e 0+e],[0 0 1 1-e 0+e 0+e],c2);patch([0 0 1 1-e 0+e 0+e],[0 1 1 1-e 1-e 0+e],c3);plot([0 1 1 0 0],[0 0 1 1 0]);disp('b');
  netsum : Sum  Inputs=2  SaturateOnIntegerOverflow=on  InputSameDT=on  OutDataTypeStr=Inherit: Same as first input
      mask Display: e = 0.06;c1 = [120 150 230]/255;c2 = ([120 150 230]+0)/383;c3 = ([120 150 230]+128)/383;patch([0 1 1 0],[0 0 1 1],c1);patch([0 1 1 1-e 1-e 0+e],[0 0 1 1-e 0+e 0+e],c2);patch([0 0 1 1-e 0+e 0+e],[0 1 1 1-e 1-e 0+e],c3);plot([0 1 1 0 0],[0 0 1 1 0]);disp('+');
  poslin : neural/Transfer Functions/poslin
      settings: SourceProductName=Deep Learning Toolbox  SourceProductBaseCode=NN
  "a{1}" : Outport  InitialOutput=[0;0;0;0;0;0;0;0;0;0;0;0;0;0;0;0;0;0;0;0;0;0;0;0;0;0;0;0;0;0;0;0;0;0;0;0;0;0;0;0;0;0;0;0;0;0;0;0;0;0]
      mask Display: e = 0.06;c1 = [120 230 180]/255;c2 = ([120 230 180]+0)/(256+128);c3 = ([120 230 180]+128)/(256+128);patch([0 1 1 0],[0 0 1 1],c1);patch([0 1 1 1-e 1-e 0+e],[0 0 1 1-e 0+e 0+e],c2);patch([0 0 1 1-e 0+e 0+e],[0 1 1 1-e 1-e 0+e],c3);plot([0 1 1 0 0],[0 0 1 1 0]);
  wires:
  poslin/1 -> "a{1}"/1
  netsum/1 -> poslin/1
  "b{1}"/1 -> netsum/2
  "IW{1,1}"/1("iz{1,1}") -> netsum/1
  Delays 1/1("pd{1,1}") -> "IW{1,1}"/1("pd{1,1}")
  "p{1}"/1 -> Delays 1/1("p{1}")

== system AcasXuClosedLoop_m_1_batch/networks/AcasXu_5_1/Layer 2 ==
  "a{1} " : Inport  PortDimensions=50
      mask Display: e = 0.06;c1 = [120 230 180]/255;c2 = ([120 230 180]+0)/(256+128);c3 = ([120 230 180]+128)/(256+128);patch([0 1 1 0],[0 0 1 1],c1);patch([0 1 1 1-e 1-e 0+e],[0 0 1 1-e 0+e 0+e],c2);patch([0 0 1 1-e 0+e 0+e],[0 1 1 1-e 1-e 0+e],c3);plot([0 1 1 0 0],[0 0 1 1 0]);
  Delays 1 : Subsystem
      mask Display: e = 0.06;c1 = [120 150 230]/255;c2 = ([120 150 230]+0)/383;c3 = ([120 150 230]+128)/383;patch([0 1 1 0],[0 0 1 1],c1);patch([0 1 1 1-e 1-e 0+e],[0 0 1 1-e 0+e 0+e],c2);patch([0 0 1 1-e 0+e 0+e],[0 1 1 1-e 1-e 0+e],c3);plot([0 1 1 0 0],[0 0 1 1 0]);disp('0');
  "LW{2,1}" : Subsystem
      mask Display: e = 0.06;c1 = [120 150 230]/255;c2 = ([120 150 230]+0)/383;c3 = ([120 150 230]+128)/383;patch([0 1 1 0],[0 0 1 1],c1);patch([0 1 1 1-e 1-e 0+e],[0 0 1 1-e 0+e 0+e],c2);patch([0 0 1 1-e 0+e 0+e],[0 1 1 1-e 1-e 0+e],c3);plot([0 1 1 0 0],[0 0 1 1 0]);disp('W');
  "b{2}" : Constant  Value=[0.69435400000000002673772314665256999433040618896484375;-0.208757999999999999118927007657475769519805908203125;-0.3371049999999999879918277656543068587779998779296875;0.395558000000000020701662606370518915355205535888671875;-1.0438400000000001011102312986622564494609832763671875;0.075501600000000002044231450781808234751224517822265625;-1.16669000000000000483169060316868126392364501953125;0.10205999999999999794386695839421008713543415069580078125;-0.2062150000000000094058094646243262104690074920654296875;-0.2491279999999999883453227766949567012488842010498046875;-0.292478999999999988990140309397247619926929473876953125;-1.1702999999999998959054892111453227698802947998046875;-1.280909999999999993036681189551018178462982177734375;-0.77106200000000002514610741854994557797908782958984375;-0.01460879999999999991067145543865990475751459598541259765625;-0.1493609999999999937703165642233216203749179840087890625;-0.76321399999999994800958802443346939980983734130859375;1.0952200000000000823519030745956115424633026123046875;0.2091240000000000043289816176184103824198246002197265625;1.4441100000000000047606363295926712453365325927734375;0.1825569999999999970530240034349844790995121002197265625;0.461874999999999980015985556747182272374629974365234375;-1.1703799999999999759126012577326036989688873291015625;0.6556729999999999503046410609385930001735687255859375;-0.05577669999999999850270882006952888332307338714599609375;-0.489686999999999983401721692644059658050537109375;1.0898399999999999199218336798367090523242950439453125;0.2116010000000000113029585691037937067449092864990234375;0.53763199999999999878497192185022868216037750244140625;-0.01333890000000000075619510653268662281334400177001953125;-1.1640200000000000546407363799517042934894561767578125;-0.414883999999999975027975551711278967559337615966796875;-0.496560999999999974630071619685622863471508026123046875;-0.0183939000000000012213785538506272132508456707000732421875;0.2301870000000000027196023211217834614217281341552734375;-0.1089299999999999990496490909208660013973712921142578125;-0.73875999999999997225330616856808774173259735107421875;0.097548899999999993948307519531226716935634613037109375;0.4301559999999999828190766493207775056362152099609375;-0.014394099999999999950350826338762999512255191802978515625;-0.0091775699999999994782573509155554347671568393707275390625;0.134299000000000001708855279503040947020053863525390625;-0.015574799999999999755573298898525536060333251953125;-0.78898999999999996912691813122364692389965057373046875;-0.146892999999999995797139717979007400572299957275390625;-0.85777300000000000768096697356668300926685333251953125;-1.260520000000000084838802649755962193012237548828125;-0.62323099999999997944399865446030162274837493896484375;-0.480572999999999972420283711471711285412311553955078125;0.85980299999999998394883959917933680117130279541015625]
      mask Display: e = 0.06;c1 = [120 150 230]/255;c2 = ([120 150 230]+0)/383;c3 = ([120 150 230]+128)/383;patch([0 1 1 0],[0 0 1 1],c1);patch([0 1 1 1-e 1-e 0+e],[0 0 1 1-e 0+e 0+e],c2);patch([0 0 1 1-e 0+e 0+e],[0 1 1 1-e 1-e 0+e],c3);plot([0 1 1 0 0],[0 0 1 1 0]);disp('b');
  netsum : Sum  Inputs=2  SaturateOnIntegerOverflow=on  InputSameDT=on  OutDataTypeStr=Inherit: Same as first input
      mask Display: e = 0.06;c1 = [120 150 230]/255;c2 = ([120 150 230]+0)/383;c3 = ([120 150 230]+128)/383;patch([0 1 1 0],[0 0 1 1],c1);patch([0 1 1 1-e 1-e 0+e],[0 0 1 1-e 0+e 0+e],c2);patch([0 0 1 1-e 0+e 0+e],[0 1 1 1-e 1-e 0+e],c3);plot([0 1 1 0 0],[0 0 1 1 0]);disp('+');
  poslin : neural/Transfer Functions/poslin
      settings: SourceProductName=Deep Learning Toolbox  SourceProductBaseCode=NN
  "a{2}" : Outport  InitialOutput=[0;0;0;0;0;0;0;0;0;0;0;0;0;0;0;0;0;0;0;0;0;0;0;0;0;0;0;0;0;0;0;0;0;0;0;0;0;0;0;0;0;0;0;0;0;0;0;0;0;0]
      mask Display: e = 0.06;c1 = [120 230 180]/255;c2 = ([120 230 180]+0)/(256+128);c3 = ([120 230 180]+128)/(256+128);patch([0 1 1 0],[0 0 1 1],c1);patch([0 1 1 1-e 1-e 0+e],[0 0 1 1-e 0+e 0+e],c2);patch([0 0 1 1-e 0+e 0+e],[0 1 1 1-e 1-e 0+e],c3);plot([0 1 1 0 0],[0 0 1 1 0]);
  wires:
  poslin/1 -> "a{2}"/1
  netsum/1 -> poslin/1
  "b{2}"/1 -> netsum/2
  "LW{2,1}"/1("lz{2,1}") -> netsum/1
  Delays 1/1("pd{2,1}") -> "LW{2,1}"/1("ad{2,1}")
  "a{1} "/1 -> Delays 1/1("p{2}")
